simulink model "pfb_float_4k_sim"  (R2018b)
settings: Solver=VariableStepDiscrete  StartTime=0.0  StopTime=8192*2^2  RelTol=1e-3
undefined symbols (not defined in the file): fft_stages
libraries: xbsIndex_r4 x16102, casper_library_misc x27, casper_library_delays x15, casper_library_sources x4, xbsReplacements_r4 x3, xps_library x1
(part 40 of 133)

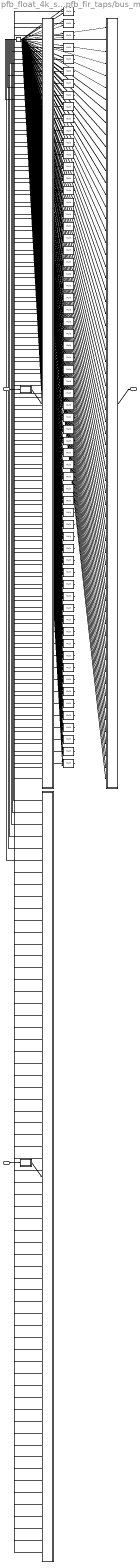
== system pfb_float_4k_sim/pfb/pfb_fir_generic/pfb_fir_taps/bus_mult ==
  a : Inport
  b : Inport  Port=2
  a*b_bussify : Subsystem  AncestorBlock=casper_library_flow_control/bus_create  mask(bus_create){inputNum=64}
      mask prompts: inputNum "Number of inputs:"
      mask init:
      | bus_create_init(gcb,...
      |     'inputNum', inputNum);
  a_debus : Subsystem  AncestorBlock=casper_library_flow_control/bus_expand  mask(bus_expand){mode=divisions of arbitrary size, outputNum=1, outputWidth=[32 32 32 32 32 32 32 32 32 32 32 32 32 32 32 32 32 32 32 32 32 32 32 32 32 32 32 32 32 32 32 32 32 32 32 32 32 32 32 32 32 32 32 32 32 32 32 32 32 32 32 32 32 32 32 32 32 32 32 32 32 32 32 32], outputBinaryPt=[0 0 0 0 0 0 0 0 0 0 0 0 0 0 0 0 0 0 0 0 0 0 0 0 0 0 0 0 0 0 0 0 0 0 0 0 0 0 0 0 0 0 0 0 0 0 0 0 0 0 0 0 0 0 0 0 0 0 0 0 0 0 0 0], outputArithmeticType=[0 0 0 0 0 0 0 0 0 0 0 0 0 0 0 0 0 0 0 0 0 0 0 0 0 0 0 0 0 0 0 0 0 0 0 0 0 0 0 0 0 0 0 0 0 0 0 0 0 0 0 0 0 0 0 0 0 0 0 0 0 0 0 0], show_format=on, outputToWorkspace=off, variablePrefix=, outputToModelAsWell=on}
      mask prompts: outputNum "Number of outputs:"; outputWidth "Output width [msb ... lsb]:"; outputBinaryPt "Output binary point position [msb ... lsb]:"; outputArithmeticType "Output arithmetic type (ufix=0, fix=1, bool=2) [msb ... lsb]:"; show_format "Print format string?"; outputToWorkspace "Output to workspace?"; variablePrefix "Variable name prefix:"; outputToModelAsWell "Output to model as well?"
      mask help:
      | Expands a 'bus' made using a bus_create or Xilinx
      | concat block.
      | The input will be divided into the specified number
      | of outputs with the specified arithmetic types.
      | Divisions are made from most significant bit.
      mask init: same as pfb_float_4k_sim/bus_expand1
      settings: AttributesFormatString=64 outputs: uf32.0,uf32.0,uf32.0,uf32.0,uf32.0,uf32.0,uf32.0,uf32.0,uf32.0,uf32.0,uf32.0,uf32.0,uf32.0,uf32.0,uf32.0,uf32.0,uf32.0,uf32.0,uf32.0,uf32.0,uf32.0,uf32.0,uf32.0,uf32.0,uf32.0,uf32.0,uf32.0,uf32.0,uf32.0,uf32.0,uf32.0,uf32.0,uf32.0,uf32.0,uf32.0,uf32.0,uf32.0,uf32.0,uf32.0,uf32.0,uf32.0,uf32.0,uf32.0,uf32.0,uf32.0,uf32.0,uf32.0,uf32.0,uf32.0,uf32.0,uf32.0,uf32.0,uf32.0,uf32.0,uf32.0,uf32.0,uf32.0,uf32.0,uf32.0,uf32.0,uf32.0,uf32.0,uf32.0,uf32.0,
      mask TypeOptions mode: divisions of equal size | divisions of arbitrary size
      mask Callback mode: bus_expand_callback();
      mask Callback outputWidth: bus_expand_callback();
      mask Callback outputToWorkspace: bus_expand_callback();
  b_debus : Subsystem  AncestorBlock=casper_library_flow_control/bus_expand  mask(bus_expand){mode=divisions of arbitrary size, outputNum=1, outputWidth=[32 32 32 32 32 32 32 32 32 32 32 32 32 32 32 32 32 32 32 32 32 32 32 32 32 32 32 32 32 32 32 32 32 32 32 32 32 32 32 32 32 32 32 32 32 32 32 32 32 32 32 32 32 32 32 32 32 32 32 32 32 32 32 32], outputBinaryPt=[0 0 0 0 0 0 0 0 0 0 0 0 0 0 0 0 0 0 0 0 0 0 0 0 0 0 0 0 0 0 0 0 0 0 0 0 0 0 0 0 0 0 0 0 0 0 0 0 0 0 0 0 0 0 0 0 0 0 0 0 0 0 0 0], outputArithmeticType=[0 0 0 0 0 0 0 0 0 0 0 0 0 0 0 0 0 0 0 0 0 0 0 0 0 0 0 0 0 0 0 0 0 0 0 0 0 0 0 0 0 0 0 0 0 0 0 0 0 0 0 0 0 0 0 0 0 0 0 0 0 0 0 0], show_format=on, outputToWorkspace=off, variablePrefix=, outputToModelAsWell=on}
      mask prompts: outputNum "Number of outputs:"; outputWidth "Output width [msb ... lsb]:"; outputBinaryPt "Output binary point position [msb ... lsb]:"; outputArithmeticType "Output arithmetic type (ufix=0, fix=1, bool=2) [msb ... lsb]:"; show_format "Print format string?"; outputToWorkspace "Output to workspace?"; variablePrefix "Variable name prefix:"; outputToModelAsWell "Output to model as well?"
      mask help:
      | Expands a 'bus' made using a bus_create or Xilinx
      | concat block.
      | The input will be divided into the specified number
      | of outputs with the specified arithmetic types.
      | Divisions are made from most significant bit.
      mask init: same as pfb_float_4k_sim/bus_expand1
      settings: AttributesFormatString=64 outputs: uf32.0,uf32.0,uf32.0,uf32.0,uf32.0,uf32.0,uf32.0,uf32.0,uf32.0,uf32.0,uf32.0,uf32.0,uf32.0,uf32.0,uf32.0,uf32.0,uf32.0,uf32.0,uf32.0,uf32.0,uf32.0,uf32.0,uf32.0,uf32.0,uf32.0,uf32.0,uf32.0,uf32.0,uf32.0,uf32.0,uf32.0,uf32.0,uf32.0,uf32.0,uf32.0,uf32.0,uf32.0,uf32.0,uf32.0,uf32.0,uf32.0,uf32.0,uf32.0,uf32.0,uf32.0,uf32.0,uf32.0,uf32.0,uf32.0,uf32.0,uf32.0,uf32.0,uf32.0,uf32.0,uf32.0,uf32.0,uf32.0,uf32.0,uf32.0,uf32.0,uf32.0,uf32.0,uf32.0,uf32.0,
      mask TypeOptions mode: divisions of equal size | divisions of arbitrary size
      mask Callback mode: bus_expand_callback();
      mask Callback outputWidth: bus_expand_callback();
      mask Callback outputToWorkspace: bus_expand_callback();
  mult{1..64} (64 alike) : xbsIndex_r4/Mult  precision=Full  arith_type="Signed  (2's comp)"  n_bits=50  bin_pt=48  quantization=Truncate  overflow=Wrap  en=off  latency=mult_latency  use_behavioral_HDL=on  opt=Speed  use_embedded=off  optimum_pipeline=off  pipeline=on  dbl_ovrd=off  use_rpm=on  placement_style=Triangular  block_type=mult
  reint_out{1..64} (64 alike) : xbsIndex_r4/Reinterpret  force_arith_type=on  arith_type=Unsigned  force_bin_pt=on  bin_pt=0  block_type=reinterpret
  reinta{1..64} (64 alike) : xbsIndex_r4/Reinterpret  force_arith_type=on  arith_type=Floating-point  force_bin_pt=on  bin_pt=24  block_type=reinterpret
  reintb{1..64} (64 alike) : xbsIndex_r4/Reinterpret  force_arith_type=on  arith_type=Floating-point  force_bin_pt=on  bin_pt=24  block_type=reinterpret
  repa : Subsystem  AncestorBlock=casper_library_bus/bus_replicate  mask(bus_replicate){replication=1, csp_latency=0, misc=off, implementation=core}
      mask prompts: replication "replication factor"; csp_latency "latency"; misc "misc support"; implementation "delay implementation"
      mask help: Output bus formed by replicating input bus a number of times
      mask init:
      | bus_replicate_init(gcb, ...
      |     'replication', replication, ...
      |     'csp_latency', csp_latency, ...
      |     'implementation', implementation, ...
      |     'misc', misc);
      mask TypeOptions implementation: core | behavioral
  repb : Subsystem  AncestorBlock=casper_library_bus/bus_replicate  mask(bus_replicate){replication=1, csp_latency=0, misc=off, implementation=core}
      mask prompts: replication "replication factor"; csp_latency "latency"; misc "misc support"; implementation "delay implementation"
      mask help: Output bus formed by replicating input bus a number of times
      mask init:
      | bus_replicate_init(gcb, ...
      |     'replication', replication, ...
      |     'csp_latency', csp_latency, ...
      |     'implementation', implementation, ...
      |     'misc', misc);
      mask TypeOptions implementation: core | behavioral
  a*b : Outport
  wires:
  a/1 -> repa/1(in)
  b/1 -> repb/1(in)
  repa/1(out) -> a_debus/1(bus_in)
  repb/1(out) -> b_debus/1(bus_in)
  a_debus/1(msb_out64) -> reinta1/1
  b_debus/1(msb_out64) -> reintb1/1
  reinta1/1 -> mult1/1
  reintb1/1 -> mult1/2
  a_debus/2(out63) -> reinta2/1
  b_debus/2(out63) -> reintb2/1
  reinta2/1 -> mult2/1
  reintb2/1 -> mult2/2
  a_debus/3(out62) -> reinta3/1
  b_debus/3(out62) -> reintb3/1
  reinta3/1 -> mult3/1
  reintb3/1 -> mult3/2
  a_debus/4(out61) -> reinta4/1
  b_debus/4(out61) -> reintb4/1
  reinta4/1 -> mult4/1
  reintb4/1 -> mult4/2
  a_debus/5(out60) -> reinta5/1
  b_debus/5(out60) -> reintb5/1
  reinta5/1 -> mult5/1
  reintb5/1 -> mult5/2
  a_debus/6(out59) -> reinta6/1
  b_debus/6(out59) -> reintb6/1
  reinta6/1 -> mult6/1
  reintb6/1 -> mult6/2
  a_debus/7(out58) -> reinta7/1
  b_debus/7(out58) -> reintb7/1
  reinta7/1 -> mult7/1
  reintb7/1 -> mult7/2
  a_debus/8(out57) -> reinta8/1
  b_debus/8(out57) -> reintb8/1
  reinta8/1 -> mult8/1
  reintb8/1 -> mult8/2
  a_debus/9(out56) -> reinta9/1
  b_debus/9(out56) -> reintb9/1
  reinta9/1 -> mult9/1
  reintb9/1 -> mult9/2
  a_debus/10(out55) -> reinta10/1
  b_debus/10(out55) -> reintb10/1
  reinta10/1 -> mult10/1
  reintb10/1 -> mult10/2
  a_debus/11(out54) -> reinta11/1
  b_debus/11(out54) -> reintb11/1
  reinta11/1 -> mult11/1
  reintb11/1 -> mult11/2
  a_debus/12(out53) -> reinta12/1
  b_debus/12(out53) -> reintb12/1
  reinta12/1 -> mult12/1
  reintb12/1 -> mult12/2
  a_debus/13(out52) -> reinta13/1
  b_debus/13(out52) -> reintb13/1
  reinta13/1 -> mult13/1
  reintb13/1 -> mult13/2
  a_debus/14(out51) -> reinta14/1
  b_debus/14(out51) -> reintb14/1
  reinta14/1 -> mult14/1
  reintb14/1 -> mult14/2
  a_debus/15(out50) -> reinta15/1
  b_debus/15(out50) -> reintb15/1
  reinta15/1 -> mult15/1
  reintb15/1 -> mult15/2
  a_debus/16(out49) -> reinta16/1
  b_debus/16(out49) -> reintb16/1
  reinta16/1 -> mult16/1
  reintb16/1 -> mult16/2
  a_debus/17(out48) -> reinta17/1
  b_debus/17(out48) -> reintb17/1
  reinta17/1 -> mult17/1
  reintb17/1 -> mult17/2
  a_debus/18(out47) -> reinta18/1
  b_debus/18(out47) -> reintb18/1
  reinta18/1 -> mult18/1
  reintb18/1 -> mult18/2
  a_debus/19(out46) -> reinta19/1
  b_debus/19(out46) -> reintb19/1
  reinta19/1 -> mult19/1
  reintb19/1 -> mult19/2
  a_debus/20(out45) -> reinta20/1
  b_debus/20(out45) -> reintb20/1
  reinta20/1 -> mult20/1
  reintb20/1 -> mult20/2
  a_debus/21(out44) -> reinta21/1
  b_debus/21(out44) -> reintb21/1
  reinta21/1 -> mult21/1
  reintb21/1 -> mult21/2
  a_debus/22(out43) -> reinta22/1
  b_debus/22(out43) -> reintb22/1
  reinta22/1 -> mult22/1
  reintb22/1 -> mult22/2
  a_debus/23(out42) -> reinta23/1
  b_debus/23(out42) -> reintb23/1
  reinta23/1 -> mult23/1
  reintb23/1 -> mult23/2
  a_debus/24(out41) -> reinta24/1
  b_debus/24(out41) -> reintb24/1
  reinta24/1 -> mult24/1
  reintb24/1 -> mult24/2
  a_debus/25(out40) -> reinta25/1
  b_debus/25(out40) -> reintb25/1
  reinta25/1 -> mult25/1
  reintb25/1 -> mult25/2
  a_debus/26(out39) -> reinta26/1
  b_debus/26(out39) -> reintb26/1
  reinta26/1 -> mult26/1
  reintb26/1 -> mult26/2
  a_debus/27(out38) -> reinta27/1
  b_debus/27(out38) -> reintb27/1
  reinta27/1 -> mult27/1
  reintb27/1 -> mult27/2
  a_debus/28(out37) -> reinta28/1
  b_debus/28(out37) -> reintb28/1
  reinta28/1 -> mult28/1
  reintb28/1 -> mult28/2
  a_debus/29(out36) -> reinta29/1
  b_debus/29(out36) -> reintb29/1
  reinta29/1 -> mult29/1
  reintb29/1 -> mult29/2
  a_debus/30(out35) -> reinta30/1
  b_debus/30(out35) -> reintb30/1
  reinta30/1 -> mult30/1
  reintb30/1 -> mult30/2
  a_debus/31(out34) -> reinta31/1
  b_debus/31(out34) -> reintb31/1
  reinta31/1 -> mult31/1
  reintb31/1 -> mult31/2
  a_debus/32(out33) -> reinta32/1
  b_debus/32(out33) -> reintb32/1
  reinta32/1 -> mult32/1
  reintb32/1 -> mult32/2
  a_debus/33(out32) -> reinta33/1
  b_debus/33(out32) -> reintb33/1
  reinta33/1 -> mult33/1
  reintb33/1 -> mult33/2
  a_debus/34(out31) -> reinta34/1
  b_debus/34(out31) -> reintb34/1
  reinta34/1 -> mult34/1
  reintb34/1 -> mult34/2
  a_debus/35(out30) -> reinta35/1
  b_debus/35(out30) -> reintb35/1
  reinta35/1 -> mult35/1
  reintb35/1 -> mult35/2
  a_debus/36(out29) -> reinta36/1
  b_debus/36(out29) -> reintb36/1
  reinta36/1 -> mult36/1
  reintb36/1 -> mult36/2
  a_debus/37(out28) -> reinta37/1
  b_debus/37(out28) -> reintb37/1
  reinta37/1 -> mult37/1
  reintb37/1 -> mult37/2
  a_debus/38(out27) -> reinta38/1
  b_debus/38(out27) -> reintb38/1
  reinta38/1 -> mult38/1
  reintb38/1 -> mult38/2
  a_debus/39(out26) -> reinta39/1
  b_debus/39(out26) -> reintb39/1
  reinta39/1 -> mult39/1
  reintb39/1 -> mult39/2
  a_debus/40(out25) -> reinta40/1
  b_debus/40(out25) -> reintb40/1
  reinta40/1 -> mult40/1
  reintb40/1 -> mult40/2
  a_debus/41(out24) -> reinta41/1
  b_debus/41(out24) -> reintb41/1
  reinta41/1 -> mult41/1
  reintb41/1 -> mult41/2
  a_debus/42(out23) -> reinta42/1
  b_debus/42(out23) -> reintb42/1
  reinta42/1 -> mult42/1
  reintb42/1 -> mult42/2
  a_debus/43(out22) -> reinta43/1
  b_debus/43(out22) -> reintb43/1
  reinta43/1 -> mult43/1
  reintb43/1 -> mult43/2
  a_debus/44(out21) -> reinta44/1
  b_debus/44(out21) -> reintb44/1
  reinta44/1 -> mult44/1
  reintb44/1 -> mult44/2
  a_debus/45(out20) -> reinta45/1
  b_debus/45(out20) -> reintb45/1
  reinta45/1 -> mult45/1
  reintb45/1 -> mult45/2
  a_debus/46(out19) -> reinta46/1
  b_debus/46(out19) -> reintb46/1
  reinta46/1 -> mult46/1
  reintb46/1 -> mult46/2
  a_debus/47(out18) -> reinta47/1
  b_debus/47(out18) -> reintb47/1
  reinta47/1 -> mult47/1
  reintb47/1 -> mult47/2
  a_debus/48(out17) -> reinta48/1
  b_debus/48(out17) -> reintb48/1
  reinta48/1 -> mult48/1
  reintb48/1 -> mult48/2
  a_debus/49(out16) -> reinta49/1
  b_debus/49(out16) -> reintb49/1
  reinta49/1 -> mult49/1
  reintb49/1 -> mult49/2
  a_debus/50(out15) -> reinta50/1
  b_debus/50(out15) -> reintb50/1
  reinta50/1 -> mult50/1
  reintb50/1 -> mult50/2
  a_debus/51(out14) -> reinta51/1
  b_debus/51(out14) -> reintb51/1
  reinta51/1 -> mult51/1
  reintb51/1 -> mult51/2
  a_debus/52(out13) -> reinta52/1
  b_debus/52(out13) -> reintb52/1
  reinta52/1 -> mult52/1
  reintb52/1 -> mult52/2
  a_debus/53(out12) -> reinta53/1
  b_debus/53(out12) -> reintb53/1
  reinta53/1 -> mult53/1
  reintb53/1 -> mult53/2
  a_debus/54(out11) -> reinta54/1
  b_debus/54(out11) -> reintb54/1
  reinta54/1 -> mult54/1
  reintb54/1 -> mult54/2
  a_debus/55(out10) -> reinta55/1
  b_debus/55(out10) -> reintb55/1
  reinta55/1 -> mult55/1
  reintb55/1 -> mult55/2
  a_debus/56(out9) -> reinta56/1
  b_debus/56(out9) -> reintb56/1
  reinta56/1 -> mult56/1
  reintb56/1 -> mult56/2
  a_debus/57(out8) -> reinta57/1
  b_debus/57(out8) -> reintb57/1
  reinta57/1 -> mult57/1
  reintb57/1 -> mult57/2
  a_debus/58(out7) -> reinta58/1
  b_debus/58(out7) -> reintb58/1
  reinta58/1 -> mult58/1
  reintb58/1 -> mult58/2
  a_debus/59(out6) -> reinta59/1
  b_debus/59(out6) -> reintb59/1
  reinta59/1 -> mult59/1
  reintb59/1 -> mult59/2
  a_debus/60(out5) -> reinta60/1
  b_debus/60(out5) -> reintb60/1
  reinta60/1 -> mult60/1
  reintb60/1 -> mult60/2
  a_debus/61(out4) -> reinta61/1
  b_debus/61(out4) -> reintb61/1
  reinta61/1 -> mult61/1
  reintb61/1 -> mult61/2
  a_debus/62(out3) -> reinta62/1
  b_debus/62(out3) -> reintb62/1
  reinta62/1 -> mult62/1
  reintb62/1 -> mult62/2
  a_debus/63(out2) -> reinta63/1
  b_debus/63(out2) -> reintb63/1
  reinta63/1 -> mult63/1
  reintb63/1 -> mult63/2
  a_debus/64(lsb_out1) -> reinta64/1
  b_debus/64(lsb_out1) -> reintb64/1
  reinta64/1 -> mult64/1
  reintb64/1 -> mult64/2
  mult1/1 -> reint_out1/1
  reint_out1/1 -> a*b_bussify/1(in1)
  mult2/1 -> reint_out2/1
  reint_out2/1 -> a*b_bussify/2(in2)
  mult3/1 -> reint_out3/1
  reint_out3/1 -> a*b_bussify/3(in3)
  mult4/1 -> reint_out4/1
  reint_out4/1 -> a*b_bussify/4(in4)
  mult5/1 -> reint_out5/1
  reint_out5/1 -> a*b_bussify/5(in5)
  mult6/1 -> reint_out6/1
  reint_out6/1 -> a*b_bussify/6(in6)
  mult7/1 -> reint_out7/1
  reint_out7/1 -> a*b_bussify/7(in7)
  mult8/1 -> reint_out8/1
  reint_out8/1 -> a*b_bussify/8(in8)
  mult9/1 -> reint_out9/1
  reint_out9/1 -> a*b_bussify/9(in9)
  mult10/1 -> reint_out10/1
  reint_out10/1 -> a*b_bussify/10(in10)
  mult11/1 -> reint_out11/1
  reint_out11/1 -> a*b_bussify/11(in11)
  mult12/1 -> reint_out12/1
  reint_out12/1 -> a*b_bussify/12(in12)
  mult13/1 -> reint_out13/1
  reint_out13/1 -> a*b_bussify/13(in13)
  mult14/1 -> reint_out14/1
  reint_out14/1 -> a*b_bussify/14(in14)
  mult15/1 -> reint_out15/1
  reint_out15/1 -> a*b_bussify/15(in15)
  mult16/1 -> reint_out16/1
  reint_out16/1 -> a*b_bussify/16(in16)
  mult17/1 -> reint_out17/1
  reint_out17/1 -> a*b_bussify/17(in17)
  mult18/1 -> reint_out18/1
  reint_out18/1 -> a*b_bussify/18(in18)
  mult19/1 -> reint_out19/1
  reint_out19/1 -> a*b_bussify/19(in19)
  mult20/1 -> reint_out20/1
  reint_out20/1 -> a*b_bussify/20(in20)
  mult21/1 -> reint_out21/1
  reint_out21/1 -> a*b_bussify/21(in21)
  mult22/1 -> reint_out22/1
  reint_out22/1 -> a*b_bussify/22(in22)
  mult23/1 -> reint_out23/1
  reint_out23/1 -> a*b_bussify/23(in23)
  mult24/1 -> reint_out24/1
  reint_out24/1 -> a*b_bussify/24(in24)
  mult25/1 -> reint_out25/1
  reint_out25/1 -> a*b_bussify/25(in25)
  mult26/1 -> reint_out26/1
  reint_out26/1 -> a*b_bussify/26(in26)
  mult27/1 -> reint_out27/1
  reint_out27/1 -> a*b_bussify/27(in27)
  mult28/1 -> reint_out28/1
  reint_out28/1 -> a*b_bussify/28(in28)
  mult29/1 -> reint_out29/1
  reint_out29/1 -> a*b_bussify/29(in29)
  mult30/1 -> reint_out30/1
  reint_out30/1 -> a*b_bussify/30(in30)
  mult31/1 -> reint_out31/1
  reint_out31/1 -> a*b_bussify/31(in31)
  mult32/1 -> reint_out32/1
  reint_out32/1 -> a*b_bussify/32(in32)
  mult33/1 -> reint_out33/1
  reint_out33/1 -> a*b_bussify/33(in33)
  mult34/1 -> reint_out34/1
  reint_out34/1 -> a*b_bussify/34(in34)
  mult35/1 -> reint_out35/1
  reint_out35/1 -> a*b_bussify/35(in35)
  mult36/1 -> reint_out36/1
  reint_out36/1 -> a*b_bussify/36(in36)
  mult37/1 -> reint_out37/1
  reint_out37/1 -> a*b_bussify/37(in37)
  mult38/1 -> reint_out38/1
  reint_out38/1 -> a*b_bussify/38(in38)
  mult39/1 -> reint_out39/1
  reint_out39/1 -> a*b_bussify/39(in39)
  mult40/1 -> reint_out40/1
  reint_out40/1 -> a*b_bussify/40(in40)
  mult41/1 -> reint_out41/1
  reint_out41/1 -> a*b_bussify/41(in41)
  mult42/1 -> reint_out42/1
  reint_out42/1 -> a*b_bussify/42(in42)
  mult43/1 -> reint_out43/1
  reint_out43/1 -> a*b_bussify/43(in43)
  mult44/1 -> reint_out44/1
  reint_out44/1 -> a*b_bussify/44(in44)
  mult45/1 -> reint_out45/1
  reint_out45/1 -> a*b_bussify/45(in45)
  mult46/1 -> reint_out46/1
  reint_out46/1 -> a*b_bussify/46(in46)
  mult47/1 -> reint_out47/1
  reint_out47/1 -> a*b_bussify/47(in47)
  mult48/1 -> reint_out48/1
  reint_out48/1 -> a*b_bussify/48(in48)
  mult49/1 -> reint_out49/1
  reint_out49/1 -> a*b_bussify/49(in49)
  mult50/1 -> reint_out50/1
  reint_out50/1 -> a*b_bussify/50(in50)
  mult51/1 -> reint_out51/1
  reint_out51/1 -> a*b_bussify/51(in51)
  mult52/1 -> reint_out52/1
  reint_out52/1 -> a*b_bussify/52(in52)
  mult53/1 -> reint_out53/1
  reint_out53/1 -> a*b_bussify/53(in53)
  mult54/1 -> reint_out54/1
  reint_out54/1 -> a*b_bussify/54(in54)
  mult55/1 -> reint_out55/1
  reint_out55/1 -> a*b_bussify/55(in55)
  mult56/1 -> reint_out56/1
  reint_out56/1 -> a*b_bussify/56(in56)
  mult57/1 -> reint_out57/1
  reint_out57/1 -> a*b_bussify/57(in57)
  mult58/1 -> reint_out58/1
  reint_out58/1 -> a*b_bussify/58(in58)
  mult59/1 -> reint_out59/1
  reint_out59/1 -> a*b_bussify/59(in59)
  mult60/1 -> reint_out60/1
  reint_out60/1 -> a*b_bussify/60(in60)
  mult61/1 -> reint_out61/1
  reint_out61/1 -> a*b_bussify/61(in61)
  mult62/1 -> reint_out62/1
  reint_out62/1 -> a*b_bussify/62(in62)
  mult63/1 -> reint_out63/1
  reint_out63/1 -> a*b_bussify/63(in63)
  mult64/1 -> reint_out64/1
  reint_out64/1 -> a*b_bussify/64(in64)
  a*b_bussify/1(bus_out) -> a*b/1

== system pfb_float_4k_sim/pfb/pfb_fir_generic/pfb_fir_taps/coeff_float_conv ==
  in : Inport
  conv{1..64} (64 alike) : xbsIndex_r4/Convert  gui_display_data_type=Fixed-point  arith_type=Floating-point  n_bits=16  bin_pt=14  float_type=Single  exp_bits=8  fraction_bits=24  quantization=Truncate  overflow=Wrap  en=off  latency=0  pipeline=off  dbl_ovrd=off  block_type=convert
  debus : Subsystem  AncestorBlock=casper_library_flow_control/bus_expand  mask(bus_expand){mode=divisions of equal size, outputNum=64, outputWidth=18, outputBinaryPt=17, outputArithmeticType=1, show_format=on, outputToWorkspace=off, variablePrefix=, outputToModelAsWell=on}
      mask prompts: outputNum "Number of outputs:"; outputWidth "Output width:"; outputBinaryPt "Output binary point position:"; outputArithmeticType "Output arithmetic type (ufix=0, fix=1, bool=2):"; show_format "Print format string?"; outputToWorkspace "Output to workspace?"; variablePrefix "Variable name prefix:"; outputToModelAsWell "Output to model as well?"
      mask help:
      | Expands a 'bus' made using a bus_create or Xilinx
      | concat block.
      | The input will be divided into the specified number
      | of outputs with the specified arithmetic types.
      | Divisions are made from most significant bit.
      mask init: same as pfb_float_4k_sim/bus_expand1
      settings: AttributesFormatString=64 outputs: f18.17,f18.17,f18.17,f18.17,f18.17,f18.17,f18.17,f18.17,f18.17,f18.17,f18.17,f18.17,f18.17,f18.17,f18.17,f18.17,f18.17,f18.17,f18.17,f18.17,f18.17,f18.17,f18.17,f18.17,f18.17,f18.17,f18.17,f18.17,f18.17,f18.17,f18.17,f18.17,f18.17,f18.17,f18.17,f18.17,f18.17,f18.17,f18.17,f18.17,f18.17,f18.17,f18.17,f18.17,f18.17,f18.17,f18.17,f18.17,f18.17,f18.17,f18.17,f18.17,f18.17,f18.17,f18.17,f18.17,f18.17,f18.17,f18.17,f18.17,f18.17,f18.17,f18.17,f18.17,
      mask TypeOptions mode: divisions of equal size | divisions of arbitrary size
      mask Callback mode: bus_expand_callback();
      mask Callback outputWidth: bus_expand_callback();
      mask Callback outputToWorkspace: bus_expand_callback();
  floatbus : Subsystem  AncestorBlock=casper_library_flow_control/bus_create  mask(bus_create){inputNum=64}
      mask prompts: inputNum "Number of inputs:"
      mask init:
      | bus_create_init(gcb,...
      |     'inputNum', inputNum);
  out : Outport
  wires:
  in/1 -> debus/1(bus_in)
  debus/1(msb_out64) -> conv1/1
  conv1/1 -> floatbus/1(in1)
  debus/2(out63) -> conv2/1
  conv2/1 -> floatbus/2(in2)
  debus/3(out62) -> conv3/1
  conv3/1 -> floatbus/3(in3)
  debus/4(out61) -> conv4/1
  conv4/1 -> floatbus/4(in4)
  debus/5(out60) -> conv5/1
  conv5/1 -> floatbus/5(in5)
  debus/6(out59) -> conv6/1
  conv6/1 -> floatbus/6(in6)
  debus/7(out58) -> conv7/1
  conv7/1 -> floatbus/7(in7)
  debus/8(out57) -> conv8/1
  conv8/1 -> floatbus/8(in8)
  debus/9(out56) -> conv9/1
  conv9/1 -> floatbus/9(in9)
  debus/10(out55) -> conv10/1
  conv10/1 -> floatbus/10(in10)
  debus/11(out54) -> conv11/1
  conv11/1 -> floatbus/11(in11)
  debus/12(out53) -> conv12/1
  conv12/1 -> floatbus/12(in12)
  debus/13(out52) -> conv13/1
  conv13/1 -> floatbus/13(in13)
  debus/14(out51) -> conv14/1
  conv14/1 -> floatbus/14(in14)
  debus/15(out50) -> conv15/1
  conv15/1 -> floatbus/15(in15)
  debus/16(out49) -> conv16/1
  conv16/1 -> floatbus/16(in16)
  debus/17(out48) -> conv17/1
  conv17/1 -> floatbus/17(in17)
  debus/18(out47) -> conv18/1
  conv18/1 -> floatbus/18(in18)
  debus/19(out46) -> conv19/1
  conv19/1 -> floatbus/19(in19)
  debus/20(out45) -> conv20/1
  conv20/1 -> floatbus/20(in20)
  debus/21(out44) -> conv21/1
  conv21/1 -> floatbus/21(in21)
  debus/22(out43) -> conv22/1
  conv22/1 -> floatbus/22(in22)
  debus/23(out42) -> conv23/1
  conv23/1 -> floatbus/23(in23)
  debus/24(out41) -> conv24/1
  conv24/1 -> floatbus/24(in24)
  debus/25(out40) -> conv25/1
  conv25/1 -> floatbus/25(in25)
  debus/26(out39) -> conv26/1
  conv26/1 -> floatbus/26(in26)
  debus/27(out38) -> conv27/1
  conv27/1 -> floatbus/27(in27)
  debus/28(out37) -> conv28/1
  conv28/1 -> floatbus/28(in28)
  debus/29(out36) -> conv29/1
  conv29/1 -> floatbus/29(in29)
  debus/30(out35) -> conv30/1
  conv30/1 -> floatbus/30(in30)
  debus/31(out34) -> conv31/1
  conv31/1 -> floatbus/31(in31)
  debus/32(out33) -> conv32/1
  conv32/1 -> floatbus/32(in32)
  debus/33(out32) -> conv33/1
  conv33/1 -> floatbus/33(in33)
  debus/34(out31) -> conv34/1
  conv34/1 -> floatbus/34(in34)
  debus/35(out30) -> conv35/1
  conv35/1 -> floatbus/35(in35)
  debus/36(out29) -> conv36/1
  conv36/1 -> floatbus/36(in36)
  debus/37(out28) -> conv37/1
  conv37/1 -> floatbus/37(in37)
  debus/38(out27) -> conv38/1
  conv38/1 -> floatbus/38(in38)
  debus/39(out26) -> conv39/1
  conv39/1 -> floatbus/39(in39)
  debus/40(out25) -> conv40/1
  conv40/1 -> floatbus/40(in40)
  debus/41(out24) -> conv41/1
  conv41/1 -> floatbus/41(in41)
  debus/42(out23) -> conv42/1
  conv42/1 -> floatbus/42(in42)
  debus/43(out22) -> conv43/1
  conv43/1 -> floatbus/43(in43)
  debus/44(out21) -> conv44/1
  conv44/1 -> floatbus/44(in44)
  debus/45(out20) -> conv45/1
  conv45/1 -> floatbus/45(in45)
  debus/46(out19) -> conv46/1
  conv46/1 -> floatbus/46(in46)
  debus/47(out18) -> conv47/1
  conv47/1 -> floatbus/47(in47)
  debus/48(out17) -> conv48/1
  conv48/1 -> floatbus/48(in48)
  debus/49(out16) -> conv49/1
  conv49/1 -> floatbus/49(in49)
  debus/50(out15) -> conv50/1
  conv50/1 -> floatbus/50(in50)
  debus/51(out14) -> conv51/1
  conv51/1 -> floatbus/51(in51)
  debus/52(out13) -> conv52/1
  conv52/1 -> floatbus/52(in52)
  debus/53(out12) -> conv53/1
  conv53/1 -> floatbus/53(in53)
  debus/54(out11) -> conv54/1
  conv54/1 -> floatbus/54(in54)
  debus/55(out10) -> conv55/1
  conv55/1 -> floatbus/55(in55)
  debus/56(out9) -> conv56/1
  conv56/1 -> floatbus/56(in56)
  debus/57(out8) -> conv57/1
  conv57/1 -> floatbus/57(in57)
  debus/58(out7) -> conv58/1
  conv58/1 -> floatbus/58(in58)
  debus/59(out6) -> conv59/1
  conv59/1 -> floatbus/59(in59)
  debus/60(out5) -> conv60/1
  conv60/1 -> floatbus/60(in60)
  debus/61(out4) -> conv61/1
  conv61/1 -> floatbus/61(in61)
  debus/62(out3) -> conv62/1
  conv62/1 -> floatbus/62(in62)
  debus/63(out2) -> conv63/1
  conv63/1 -> floatbus/63(in63)
  debus/64(lsb_out1) -> conv64/1
  conv64/1 -> floatbus/64(in64)
  floatbus/1(bus_out) -> out/1
